simulink library "inp"  (Simulink 8.2)
settings: Solver=ode45  SolverMode=SingleTasking  StartTime=0.0  StopTime=10.0  RelTol=1e-3
undefined symbols (not defined in the file or in its folder): C_TICK_TIME_S, ENUM_FAULTSTATE_FAIL, ENUM_FAULTSTATE_INDETERMINATE, ENUM_FAULTSTATE_PASS, ENUM_KEYSWITCH_ON, FALSE, KINP_ABS1CANCommuOk_flg_ovrdflg, KINP_ABS1CANCommuOk_flg_ovrdval, KINP_ABS1CANPeriodTime_s, KINP_ABS1CANRolCntOk_flg_ovrdflg, KINP_ABS1CANRolCntOk_flg_ovrdval, KINP_ABS2CANChkSumOk_flg_ovrdflg, KINP_ABS2CANChkSumOk_flg_ovrdval, KINP_ABS2CANCommuOk_flg_ovrdflg, KINP_ABS2CANCommuOk_flg_ovrdval, KINP_ABS2CANPeriodTime_s, KINP_ABS2CANRolCntOk_flg_ovrdflg, KINP_ABS2CANRolCntOk_flg_ovrdval, KINP_ABS3CANCommuOk_flg_ovrdflg, KINP_ABS3CANCommuOk_flg_ovrdval, KINP_ABS4CANCommuOk_flg_ovrdflg, KINP_ABS4CANCommuOk_flg_ovrdval, KINP_ABSActive_flg_defval, KINP_ABSActive_flg_invalid, KINP_ABSActive_flg_ovrdflg, KINP_ABSActive_flg_ovrdval, KINP_ABSActive_flg_setvalid, KINP_ABSActive_flg_srvcflg, KINP_ABSActive_flg_srvcval, KINP_ABSCANAlive_flg_ovrdflg, KINP_ABSCANAlive_flg_ovrdval, KINP_ABSCANCommuOk_flg_ovrdflg, KINP_ABSCANCommuOk_flg_ovrdval, KINP_ABSCANValidOk_flg_ovrdflg, KINP_ABSCANValidOk_flg_ovrdval, KINP_ABSFault_flg_defval, KINP_ABSFault_flg_invalid, KINP_ABSFault_flg_ovrdflg, KINP_ABSFault_flg_ovrdval, KINP_ABSFault_flg_setvalid, KINP_ABSFault_flg_srvcflg, KINP_ABSFault_flg_srvcval, KINP_AC1CANCommuOk_flg_ovrdflg, KINP_AC1CANCommuOk_flg_ovrdval, KINP_ACCANAlive_flg_ovrdflg, KINP_ACCANAlive_flg_ovrdval, KINP_ACCANCommuOk_flg_ovrdflg, KINP_ACCANCommuOk_flg_ovrdval, KINP_ACCANValidOk_flg_ovrdflg, KINP_ACCANValidOk_flg_ovrdval, KINP_ACCCancelH_flg_defval, KINP_ACCCancelH_flg_invalid, KINP_ACCCancelH_flg_ovrdflg, KINP_ACCCancelH_flg_ovrdval, KINP_ACCCancelH_flg_setvalid, KINP_ACCCancelH_flg_srvcflg, KINP_ACCCancelH_flg_srvcval, KINP_AccelPedalPosn_pct_defval, KINP_AccelPedalPosn_pct_gain, KINP_AccelPedalPosn_pct_invalid ... (+1866)
libraries: inp x192, hcu_lib x34, simulink x33
(part 18 of 21)

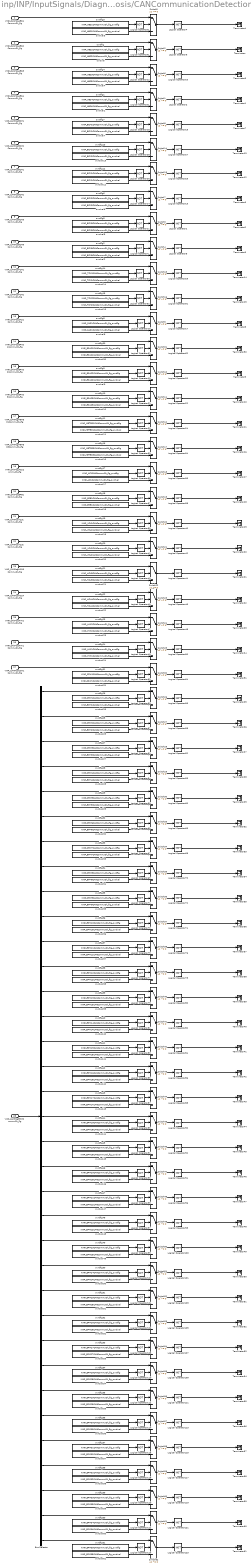
== system inp/INP/InputSignals/Diagnosis/CANCommunicationDetection_byID ==
  VINP_CANMsgABS1CommuOk_flg : Inport
  VINP_CANMsgABS2CommuOk_flg : Inport  Port=2
  VINP_CANMsgABS3CommuOk_flg : Inport  Port=3
  VINP_CANMsgABS4CommuOk_flg : Inport  Port=4
  VINP_CANMsgBCM1CommuOk_flg : Inport  Port=5
  VINP_CANMsgBCM2CommuOk_flg : Inport  Port=6
  VINP_CANMsgECM1CommuOk_flg : Inport  Port=7
  VINP_CANMsgECM2CommuOk_flg : Inport  Port=8
  VINP_CANMsgECM3CommuOk_flg : Inport  Port=9
  VINP_CANMsgECM9CommuOk_flg : Inport  Port=10
  VINP_CANMsgTCM1CommuOk_flg : Inport  Port=11
  VINP_CANMsgTCM2CommuOk_flg : Inport  Port=12
  VINP_CANMsgSAS1CommuOk_flg : Inport  Port=13
  VINP_CANMsgEPAS1CommuOk_flg : Inport  Port=14
  VINP_CANMsgEPAS2CommuOk_flg : Inport  Port=15
  VINP_CANMsgEPAS3CommuOk_flg : Inport  Port=16
  VINP_CANMsgMETER1CommuOk_flg : Inport  Port=17
  VINP_CANMsgMETER3CommuOk_flg : Inport  Port=18
  VINP_CANMsgAC1CommuOk_flg : Inport  Port=19
  VINP_CANMsgEPB1CommuOk_flg : Inport  Port=20
  VINP_CANMsgVCU1CommuOk_flg : Inport  Port=21
  VINP_CANMsgVCU2CommuOk_flg : Inport  Port=22
  VINP_CANMsgHCU3CommuOk_flg : Inport  Port=23
  VINP_CANMsgHCU4CommuOk_flg : Inport  Port=24
  VINP_CANMsgHMI2CommuOk_flg : Inport  Port=25
  VINP_CANMsgHMI1CommuOk_flg : Inport  Port=26
  VINP_CANMsgCSW1CommuOk_flg : Inport  Port=27
  VINP_CANMsgEPMCommuOk_flg : Inport  Port=28
  Bus Selector : BusSelector  OutputSignals=VINP_CANMsgEPM1CommuOk_flg,VINP_CANMsgEPM2CommuOk_flg,VINP_CANMsgEPM3CommuOk_flg,VINP_CANMsgEPM4CommuOk_flg,VINP_CANMsgEPM5CommuOk_flg,VINP_CANMsgEPM6CommuOk_flg,VINP_CANMsgEPM7CommuOk_flg,VINP_CANMsgEPM8CommuOk_flg,VINP_CANMsgEPM9CommuOk_flg,VINP_CANMsgEPM10CommuOk_flg,VINP_CANMsgEPM11CommuOk_flg,VINP_CANMsgEPM12CommuOk_flg,VINP_CANMsgEPM13CommuOk_flg,VINP_CANMsgEPM14CommuOk_flg,VINP_CANMsgEPM15CommuOk_flg,VINP_CANMsgEPM16CommuOk_flg,VINP_CANMsgEPM17CommuOk_flg,VINP_CANMsgEPM18CommuOk_flg,VINP_CANMsgEPM19CommuOk_flg,VINP_CANMsgEPM20CommuOk_flg,VINP_CANMsgEPM21CommuOk_flg,VINP_CANMsgEPM22CommuOk_flg,VINP_CANMsgEPM23CommuOk_flg,VINP_CANMsgEPM24CommuOk_flg,VINP_CANMsgEPM25CommuOk_flg,VINP_CANMsgEPM26CommuOk_flg,VINP_CANMsgEPM27CommuOk_flg,VINP_CANMsgEPM28CommuOk_flg,VINP_CANMsgEPM29CommuOk_flg,VINP_CANMsgEPM30CommuOk_flg,VINP_CANMsgEPM31CommuOk_flg,VINP_CANMsgEPM32CommuOk_flg,VINP_CANMsgEPM33CommuOk_flg,VINP_CANMsgEPM34CommuOk_flg,VINP_CANMsgEPM35CommuOk_flg
  Logical Operator{1..124} (124 alike) : Logic  Operator=NOT  AllPortsSameDT=off  OutDataTypeStr=boolean
  Switch{1..62} (62 alike) : Switch  Criteria=u2 ~= 0
  Terminator{,1..61} (62 alike) : Terminator
  ovrdflg : Constant  Value=KINP_ABS2CANCommuOk_flg_ovrdflg
  ovrdflg1 : Constant  Value=KINP_ABS4CANCommuOk_flg_ovrdflg
  ovrdflg10 : Constant  Value=KINP_EPAS3CANCommuOk_flg_ovrdflg
  ovrdflg11 : Constant  Value=KINP_METER1CANCommuOk_flg_ovrdflg
  ovrdflg12 : Constant  Value=KINP_ECM1CANCommuOk_flg_ovrdflg
  ovrdflg13 : Constant  Value=KINP_BCM2CANCommuOk_flg_ovrdflg
  ovrdflg14 : Constant  Value=KINP_TCM1CANCommuOk_flg_ovrdflg
  ovrdflg15 : Constant  Value=KINP_TCM2CANCommuOk_flg_ovrdflg
  ovrdflg16 : Constant  Value=KINP_METER3CANCommuOk_flg_ovrdflg
  ovrdflg17 : Constant  Value=KINP_AC1CANCommuOk_flg_ovrdflg
  ovrdflg18 : Constant  Value=KINP_EPB1CANCommuOk_flg_ovrdflg
  ovrdflg19 : Constant  Value=KINP_VCU1CANCommuOk_flg_ovrdflg
  ovrdflg2 : Constant  Value=KINP_ECM9CANCommuOk_flg_ovrdflg
  ovrdflg20 : Constant  Value=KINP_VCU2CANCommuOk_flg_ovrdflg
  ovrdflg21 : Constant  Value=KINP_HCU3CANCommuOk_flg_ovrdflg
  ovrdflg22 : Constant  Value=KINP_HCU4CANCommuOk_flg_ovrdflg
  ovrdflg23 : Constant  Value=KINP_HMI2CANCommuOk_flg_ovrdflg
  ovrdflg24 : Constant  Value=KINP_HMI1CANCommuOk_flg_ovrdflg
  ovrdflg25 : Constant  Value=KINP_EPM2CANCommuOk_flg_ovrdflg
  ovrdflg26 : Constant  Value=KINP_EPM1CANCommuOk_flg_ovrdflg
  ovrdflg27 : Constant  Value=KINP_EPM3CANCommuOk_flg_ovrdflg
  ovrdflg28 : Constant  Value=KINP_EPM4CANCommuOk_flg_ovrdflg
  ovrdflg29 : Constant  Value=KINP_EPM5CANCommuOk_flg_ovrdflg
  ovrdflg3 : Constant  Value=KINP_ABS1CANCommuOk_flg_ovrdflg
  ovrdflg30 : Constant  Value=KINP_EPAS1CANCommuOk_flg_ovrdflg
  ovrdflg31 : Constant  Value=KINP_CSW1CANCommuOk_flg_ovrdflg
  ovrdflg32 : Constant  Value=KINP_EPM6CANCommuOk_flg_ovrdflg
  ovrdflg33 : Constant  Value=KINP_EPM7CANCommuOk_flg_ovrdflg
  ovrdflg34 : Constant  Value=KINP_EPM8CANCommuOk_flg_ovrdflg
  ovrdflg35 : Constant  Value=KINP_EPM9CANCommuOk_flg_ovrdflg
  ovrdflg36 : Constant  Value=KINP_EPM10CANCommuOk_flg_ovrdflg
  ovrdflg37 : Constant  Value=KINP_EPM11CANCommuOk_flg_ovrdflg
  ovrdflg38 : Constant  Value=KINP_EPM12CANCommuOk_flg_ovrdflg
  ovrdflg39 : Constant  Value=KINP_EPM13CANCommuOk_flg_ovrdflg
  ovrdflg4 : Constant  Value=KINP_ABS3CANCommuOk_flg_ovrdflg
  ovrdflg40 : Constant  Value=KINP_EPM14CANCommuOk_flg_ovrdflg
  ovrdflg41 : Constant  Value=KINP_EPM15CANCommuOk_flg_ovrdflg
  ovrdflg42 : Constant  Value=KINP_EPM16CANCommuOk_flg_ovrdflg
  ovrdflg43 : Constant  Value=KINP_EPM17CANCommuOk_flg_ovrdflg
  ovrdflg44 : Constant  Value=KINP_EPM18CANCommuOk_flg_ovrdflg
  ovrdflg45 : Constant  Value=KINP_EPM19CANCommuOk_flg_ovrdflg
  ovrdflg46 : Constant  Value=KINP_EPM20CANCommuOk_flg_ovrdflg
  ovrdflg47 : Constant  Value=KINP_EPM21CANCommuOk_flg_ovrdflg
  ovrdflg48 : Constant  Value=KINP_EPM22CANCommuOk_flg_ovrdflg
  ovrdflg49 : Constant  Value=KINP_EPM23CANCommuOk_flg_ovrdflg
  ovrdflg5 : Constant  Value=KINP_ECM3CANCommuOk_flg_ovrdflg
  ovrdflg50 : Constant  Value=KINP_EPM24CANCommuOk_flg_ovrdflg
  ovrdflg51 : Constant  Value=KINP_EPM25CANCommuOk_flg_ovrdflg
  ovrdflg52 : Constant  Value=KINP_EPM26CANCommuOk_flg_ovrdflg
  ovrdflg53 : Constant  Value=KINP_EPM27CANCommuOk_flg_ovrdflg
  ovrdflg54 : Constant  Value=KINP_EPM28CANCommuOk_flg_ovrdflg
  ovrdflg55 : Constant  Value=KINP_EPM29CANCommuOk_flg_ovrdflg
  ovrdflg56 : Constant  Value=KINP_EPM30CANCommuOk_flg_ovrdflg
  ovrdflg57 : Constant  Value=KINP_EPM31CANCommuOk_flg_ovrdflg
  ovrdflg58 : Constant  Value=KINP_EPM32CANCommuOk_flg_ovrdflg
  ovrdflg59 : Constant  Value=KINP_EPM33CANCommuOk_flg_ovrdflg
  ovrdflg6 : Constant  Value=KINP_ECM2CANCommuOk_flg_ovrdflg
  ovrdflg60 : Constant  Value=KINP_EPM34CANCommuOk_flg_ovrdflg
  ovrdflg61 : Constant  Value=KINP_EPM35CANCommuOk_flg_ovrdflg
  ovrdflg7 : Constant  Value=KINP_BCM1CANCommuOk_flg_ovrdflg
  ovrdflg8 : Constant  Value=KINP_SAS1CANCommuOk_flg_ovrdflg
  ovrdflg9 : Constant  Value=KINP_EPAS2CANCommuOk_flg_ovrdflg
  ovrdval : Constant  Value=KINP_ABS2CANCommuOk_flg_ovrdval
  ovrdval1 : Constant  Value=KINP_ABS4CANCommuOk_flg_ovrdval
  ovrdval10 : Constant  Value=KINP_EPAS3CANCommuOk_flg_ovrdval
  ovrdval11 : Constant  Value=KINP_METER1CANCommuOk_flg_ovrdval
  ovrdval12 : Constant  Value=KINP_ECM1CANCommuOk_flg_ovrdval
  ovrdval13 : Constant  Value=KINP_BCM2CANCommuOk_flg_ovrdval
  ovrdval14 : Constant  Value=KINP_TCM1CANCommuOk_flg_ovrdval
  ovrdval15 : Constant  Value=KINP_TCM2CANCommuOk_flg_ovrdval
  ovrdval16 : Constant  Value=KINP_METER3CANCommuOk_flg_ovrdval
  ovrdval17 : Constant  Value=KINP_AC1CANCommuOk_flg_ovrdval
  ovrdval18 : Constant  Value=KINP_EPB1CANCommuOk_flg_ovrdval
  ovrdval19 : Constant  Value=KINP_VCU1CANCommuOk_flg_ovrdval
  ovrdval2 : Constant  Value=KINP_ECM9CANCommuOk_flg_ovrdval
  ovrdval20 : Constant  Value=KINP_VCU2CANCommuOk_flg_ovrdval
  ovrdval21 : Constant  Value=KINP_HCU3CANCommuOk_flg_ovrdval
  ovrdval22 : Constant  Value=KINP_HCU4CANCommuOk_flg_ovrdval
  ovrdval23 : Constant  Value=KINP_HMI2CANCommuOk_flg_ovrdval
  ovrdval24 : Constant  Value=KINP_HMI1CANCommuOk_flg_ovrdval
  ovrdval25 : Constant  Value=KINP_EPM2CANCommuOk_flg_ovrdval
  ovrdval26 : Constant  Value=KINP_EPM1CANCommuOk_flg_ovrdval
  ovrdval27 : Constant  Value=KINP_EPM3CANCommuOk_flg_ovrdval
  ovrdval28 : Constant  Value=KINP_EPM4CANCommuOk_flg_ovrdval
  ovrdval29 : Constant  Value=KINP_EPM5CANCommuOk_flg_ovrdval
  ovrdval3 : Constant  Value=KINP_ABS1CANCommuOk_flg_ovrdval
  ovrdval30 : Constant  Value=KINP_EPAS1CANCommuOk_flg_ovrdval
  ovrdval31 : Constant  Value=KINP_CSW1CANCommuOk_flg_ovrdval
  ovrdval32 : Constant  Value=KINP_EPM6CANCommuOk_flg_ovrdval
  ovrdval33 : Constant  Value=KINP_EPM7CANCommuOk_flg_ovrdval
  ovrdval34 : Constant  Value=KINP_EPM8CANCommuOk_flg_ovrdval
  ovrdval35 : Constant  Value=KINP_EPM9CANCommuOk_flg_ovrdval
  ovrdval36 : Constant  Value=KINP_EPM10CANCommuOk_flg_ovrdval
  ovrdval37 : Constant  Value=KINP_EPM11CANCommuOk_flg_ovrdval
  ovrdval38 : Constant  Value=KINP_EPM12CANCommuOk_flg_ovrdval
  ovrdval39 : Constant  Value=KINP_EPM13CANCommuOk_flg_ovrdval
  ovrdval4 : Constant  Value=KINP_ABS3CANCommuOk_flg_ovrdval
  ovrdval40 : Constant  Value=KINP_EPM14CANCommuOk_flg_ovrdval
  ovrdval41 : Constant  Value=KINP_EPM15CANCommuOk_flg_ovrdval
  ovrdval42 : Constant  Value=KINP_EPM16CANCommuOk_flg_ovrdval
  ovrdval43 : Constant  Value=KINP_EPM17CANCommuOk_flg_ovrdval
  ovrdval44 : Constant  Value=KINP_EPM18CANCommuOk_flg_ovrdval
  ovrdval45 : Constant  Value=KINP_EPM19CANCommuOk_flg_ovrdval
  ovrdval46 : Constant  Value=KINP_EPM20CANCommuOk_flg_ovrdval
  ovrdval47 : Constant  Value=KINP_EPM21CANCommuOk_flg_ovrdval
  ovrdval48 : Constant  Value=KINP_EPM22CANCommuOk_flg_ovrdval
  ovrdval49 : Constant  Value=KINP_EPM23CANCommuOk_flg_ovrdval
  ovrdval5 : Constant  Value=KINP_ECM3CANCommuOk_flg_ovrdval
  ovrdval50 : Constant  Value=KINP_EPM24CANCommuOk_flg_ovrdval
  ovrdval51 : Constant  Value=KINP_EPM25CANCommuOk_flg_ovrdval
  ovrdval52 : Constant  Value=KINP_EPM26CANCommuOk_flg_ovrdval
  ovrdval53 : Constant  Value=KINP_EPM27CANCommuOk_flg_ovrdval
  ovrdval54 : Constant  Value=KINP_EPM28CANCommuOk_flg_ovrdval
  ovrdval55 : Constant  Value=KINP_EPM29CANCommuOk_flg_ovrdval
  ovrdval56 : Constant  Value=KINP_EPM30CANCommuOk_flg_ovrdval
  ovrdval57 : Constant  Value=KINP_EPM31CANCommuOk_flg_ovrdval
  ovrdval58 : Constant  Value=KINP_EPM32CANCommuOk_flg_ovrdval
  ovrdval59 : Constant  Value=KINP_EPM33CANCommuOk_flg_ovrdval
  ovrdval6 : Constant  Value=KINP_ECM2CANCommuOk_flg_ovrdval
  ovrdval60 : Constant  Value=KINP_EPM34CANCommuOk_flg_ovrdval
  ovrdval61 : Constant  Value=KINP_EPM35CANCommuOk_flg_ovrdval
  ovrdval7 : Constant  Value=KINP_BCM1CANCommuOk_flg_ovrdval
  ovrdval8 : Constant  Value=KINP_SAS1CANCommuOk_flg_ovrdval
  ovrdval9 : Constant  Value=KINP_EPAS2CANCommuOk_flg_ovrdval
  wires:
  Logical Operator1/1 -> Terminator2/1  "VINP_ECM3CANCommuFault_flg"
  Logical Operator6/1 -> Terminator/1  "VINP_ABS2CANCommuFault_flg"
  Logical Operator11/1 -> Switch3/2
  Logical Operator8/1 -> Switch2/2
  ovrdval5/1 -> Switch3/3
  ovrdflg5/1 -> Logical Operator11/1
  ovrdval/1 -> Switch2/3
  ovrdflg/1 -> Logical Operator8/1
  Switch3/1 -> Logical Operator1/1
  Switch2/1 -> Logical Operator6/1
  VINP_CANMsgABS2CommuOk_flg/1 -> Switch2/1
  VINP_CANMsgECM3CommuOk_flg/1 -> Switch3/1
  Logical Operator2/1 -> Terminator1/1  "VINP_ABS4CANCommuFault_flg"
  Logical Operator3/1 -> Switch1/2
  ovrdval1/1 -> Switch1/3
  ovrdflg1/1 -> Logical Operator3/1
  Switch1/1 -> Logical Operator2/1
  VINP_CANMsgABS4CommuOk_flg/1 -> Switch1/1
  Logical Operator5/1 -> Terminator3/1  "VINP_ECM9CANCommuFault_flg"
  Logical Operator7/1 -> Switch4/2
  ovrdval2/1 -> Switch4/3
  ovrdflg2/1 -> Logical Operator7/1
  Switch4/1 -> Logical Operator5/1
  VINP_CANMsgECM9CommuOk_flg/1 -> Switch4/1
  Logical Operator17/1 -> Terminator8/1  "VINP_SAS1CANCommuFault_flg"
  Logical Operator16/1 -> Switch9/2
  ovrdval8/1 -> Switch9/3
  ovrdflg8/1 -> Logical Operator16/1
  Switch9/1 -> Logical Operator17/1
  VINP_CANMsgSAS1CommuOk_flg/1 -> Switch9/1
  Logical Operator20/1 -> Terminator9/1  "VINP_EPAS2CANCommuFault_flg"
  Logical Operator19/1 -> Switch10/2
  ovrdval9/1 -> Switch10/3
  ovrdflg9/1 -> Logical Operator19/1
  Switch10/1 -> Logical Operator20/1
  VINP_CANMsgEPAS2CommuOk_flg/1 -> Switch10/1
  Logical Operator22/1 -> Terminator10/1  "VINP_EPAS3CANCommuFault_flg"
  Logical Operator21/1 -> Switch11/2
  ovrdval10/1 -> Switch11/3
  ovrdflg10/1 -> Logical Operator21/1
  Switch11/1 -> Logical Operator22/1
  VINP_CANMsgEPAS3CommuOk_flg/1 -> Switch11/1
  Logical Operator24/1 -> Terminator11/1  "VINP_METER1CANCommuFault_flg"
  Logical Operator23/1 -> Switch12/2
  ovrdval11/1 -> Switch12/3
  ovrdflg11/1 -> Logical Operator23/1
  Switch12/1 -> Logical Operator24/1
  VINP_CANMsgMETER1CommuOk_flg/1 -> Switch12/1
  Logical Operator25/1 -> Terminator12/1  "VINP_ECM1CANCommuFault_flg"
  Logical Operator26/1 -> Switch13/2
  ovrdval12/1 -> Switch13/3
  ovrdflg12/1 -> Logical Operator26/1
  Switch13/1 -> Logical Operator25/1
  VINP_CANMsgECM1CommuOk_flg/1 -> Switch13/1
  Logical Operator4/1 -> Terminator4/1  "VINP_ABS1CANCommuFault_flg"
  Logical Operator9/1 -> Switch5/2
  ovrdval3/1 -> Switch5/3
  ovrdflg3/1 -> Logical Operator9/1
  Switch5/1 -> Logical Operator4/1
  VINP_CANMsgABS1CommuOk_flg/1 -> Switch5/1
  Logical Operator10/1 -> Terminator5/1  "VINP_ABS3CANCommuFault_flg"
  Logical Operator12/1 -> Switch6/2
  ovrdval4/1 -> Switch6/3
  ovrdflg4/1 -> Logical Operator12/1
  Switch6/1 -> Logical Operator10/1
  VINP_CANMsgABS3CommuOk_flg/1 -> Switch6/1
  Logical Operator13/1 -> Terminator6/1  "VINP_ECM2CANCommuFault_flg"
  Logical Operator14/1 -> Switch7/2
  ovrdval6/1 -> Switch7/3
  ovrdflg6/1 -> Logical Operator14/1
  Switch7/1 -> Logical Operator13/1
  VINP_CANMsgECM2CommuOk_flg/1 -> Switch7/1
  Logical Operator15/1 -> Terminator7/1  "VINP_BCM1CANCommuFault_flg"
  Logical Operator18/1 -> Switch8/2
  ovrdval7/1 -> Switch8/3
  ovrdflg7/1 -> Logical Operator18/1
  Switch8/1 -> Logical Operator15/1
  VINP_CANMsgBCM1CommuOk_flg/1 -> Switch8/1
  Logical Operator27/1 -> Terminator13/1  "VINP_BCM2CANCommuFault_flg"
  Logical Operator28/1 -> Switch14/2
  ovrdval13/1 -> Switch14/3
  ovrdflg13/1 -> Logical Operator28/1
  Switch14/1 -> Logical Operator27/1
  VINP_CANMsgBCM2CommuOk_flg/1 -> Switch14/1
  Logical Operator29/1 -> Terminator14/1  "VINP_TCM1CANCommuFault_flg"
  Logical Operator30/1 -> Switch15/2
  ovrdval14/1 -> Switch15/3
  ovrdflg14/1 -> Logical Operator30/1
  Switch15/1 -> Logical Operator29/1
  VINP_CANMsgTCM1CommuOk_flg/1 -> Switch15/1
  Logical Operator31/1 -> Terminator15/1  "VINP_TCM2CANCommuFault_flg"
  Logical Operator32/1 -> Switch16/2
  ovrdval15/1 -> Switch16/3
  ovrdflg15/1 -> Logical Operator32/1
  Switch16/1 -> Logical Operator31/1
  VINP_CANMsgTCM2CommuOk_flg/1 -> Switch16/1
  Logical Operator34/1 -> Terminator16/1  "VINP_METER3CANCommuFault_flg"
  Logical Operator33/1 -> Switch17/2
  ovrdval16/1 -> Switch17/3
  ovrdflg16/1 -> Logical Operator33/1
  Switch17/1 -> Logical Operator34/1
  VINP_CANMsgMETER3CommuOk_flg/1 -> Switch17/1
  Logical Operator36/1 -> Terminator17/1  "VINP_AC1CANCommuFault_flg"
  Logical Operator35/1 -> Switch18/2
  ovrdval17/1 -> Switch18/3
  ovrdflg17/1 -> Logical Operator35/1
  Switch18/1 -> Logical Operator36/1
  VINP_CANMsgAC1CommuOk_flg/1 -> Switch18/1
  Logical Operator38/1 -> Terminator18/1  "VINP_EPB1CANCommuFault_flg"
  Logical Operator37/1 -> Switch19/2
  ovrdval18/1 -> Switch19/3
  ovrdflg18/1 -> Logical Operator37/1
  Switch19/1 -> Logical Operator38/1
  VINP_CANMsgEPB1CommuOk_flg/1 -> Switch19/1
  Logical Operator40/1 -> Terminator19/1  "VINP_VCU1CANCommuFault_flg"
  Logical Operator39/1 -> Switch20/2
  ovrdval19/1 -> Switch20/3
  ovrdflg19/1 -> Logical Operator39/1
  Switch20/1 -> Logical Operator40/1
  VINP_CANMsgVCU1CommuOk_flg/1 -> Switch20/1
  Logical Operator42/1 -> Terminator20/1  "VINP_VCU2CANCommuFault_flg"
  Logical Operator41/1 -> Switch21/2
  ovrdval20/1 -> Switch21/3
  ovrdflg20/1 -> Logical Operator41/1
  Switch21/1 -> Logical Operator42/1
  VINP_CANMsgVCU2CommuOk_flg/1 -> Switch21/1
  Logical Operator44/1 -> Terminator21/1  "VINP_HCU3CANCommuFault_flg"
  Logical Operator43/1 -> Switch22/2
  ovrdval21/1 -> Switch22/3
  ovrdflg21/1 -> Logical Operator43/1
  Switch22/1 -> Logical Operator44/1
  VINP_CANMsgHCU3CommuOk_flg/1 -> Switch22/1
  Logical Operator46/1 -> Terminator22/1  "VINP_HCU4CANCommuFault_flg"
  Logical Operator45/1 -> Switch23/2
  ovrdval22/1 -> Switch23/3
  ovrdflg22/1 -> Logical Operator45/1
  Switch23/1 -> Logical Operator46/1
  VINP_CANMsgHCU4CommuOk_flg/1 -> Switch23/1
  Logical Operator48/1 -> Terminator23/1  "VINP_HMI2CANCommuFault_flg"
  Logical Operator47/1 -> Switch24/2
  ovrdval23/1 -> Switch24/3
  ovrdflg23/1 -> Logical Operator47/1
  Switch24/1 -> Logical Operator48/1
  VINP_CANMsgHMI2CommuOk_flg/1 -> Switch24/1
  Logical Operator50/1 -> Terminator24/1  "VINP_HMI1CANCommuFault_flg"
  Logical Operator49/1 -> Switch25/2
  ovrdval24/1 -> Switch25/3
  ovrdflg24/1 -> Logical Operator49/1
  Switch25/1 -> Logical Operator50/1
  VINP_CANMsgHMI1CommuOk_flg/1 -> Switch25/1
  Logical Operator56/1 -> Terminator25/1  "VINP_EPM1CANCommuFault_flg"
  Logical Operator53/1 -> Switch26/2
  ovrdval26/1 -> Switch26/3
  ovrdflg26/1 -> Logical Operator53/1
  Switch26/1 -> Logical Operator56/1
  VINP_CANMsgEPMCommuOk_flg/1 -> Bus Selector/1
  Logical Operator58/1 -> Terminator26/1  "VINP_EPM2CANCommuFault_flg"
  Logical Operator57/1 -> Switch27/2
  ovrdval25/1 -> Switch27/3
  ovrdflg25/1 -> Logical Operator57/1
  Switch27/1 -> Logical Operator58/1
  Logical Operator52/1 -> Terminator27/1  "VINP_EPM3CANCommuFault_flg"
  Logical Operator51/1 -> Switch28/2
  ovrdval27/1 -> Switch28/3
  ovrdflg27/1 -> Logical Operator51/1
  Switch28/1 -> Logical Operator52/1
  Logical Operator55/1 -> Terminator28/1  "VINP_EPM4CANCommuFault_flg"
  Logical Operator54/1 -> Switch29/2
  ovrdval28/1 -> Switch29/3
  ovrdflg28/1 -> Logical Operator54/1
  Switch29/1 -> Logical Operator55/1
  Logical Operator60/1 -> Terminator29/1  "VINP_EPM5CANCommuFault_flg"
  Logical Operator59/1 -> Switch30/2
  ovrdval29/1 -> Switch30/3
  ovrdflg29/1 -> Logical Operator59/1
  Switch30/1 -> Logical Operator60/1
  Bus Selector/5 -> Switch30/1  "<VINP_CANMsgEPM5CommuOk_flg>"
  Logical Operator62/1 -> Terminator30/1  "VINP_EPAS1CANCommuFault_flg"
  Logical Operator61/1 -> Switch31/2
  ovrdval30/1 -> Switch31/3
  ovrdflg30/1 -> Logical Operator61/1
  Switch31/1 -> Logical Operator62/1
  VINP_CANMsgEPAS1CommuOk_flg/1 -> Switch31/1
  Logical Operator64/1 -> Terminator31/1  "VINP_CSW1CANCommuFault_flg"
  Logical Operator63/1 -> Switch32/2
  ovrdval31/1 -> Switch32/3
  ovrdflg31/1 -> Logical Operator63/1
  Switch32/1 -> Logical Operator64/1
  VINP_CANMsgCSW1CommuOk_flg/1 -> Switch32/1
  Bus Selector/1 -> Switch26/1  "<VINP_CANMsgEPM1CommuOk_flg>"
  Bus Selector/2 -> Switch27/1  "<VINP_CANMsgEPM2CommuOk_flg>"
  Bus Selector/3 -> Switch28/1  "<VINP_CANMsgEPM3CommuOk_flg>"
  Bus Selector/4 -> Switch29/1  "<VINP_CANMsgEPM4CommuOk_flg>"
  Logical Operator66/1 -> Terminator32/1  "VINP_EPM6CANCommuFault_flg"
  Logical Operator65/1 -> Switch33/2
  ovrdval32/1 -> Switch33/3
  ovrdflg32/1 -> Logical Operator65/1
  Switch33/1 -> Logical Operator66/1
  Bus Selector/6 -> Switch33/1  "<VINP_CANMsgEPM6CommuOk_flg>"
  Logical Operator68/1 -> Terminator33/1  "VINP_EPM7CANCommuFault_flg"
  Logical Operator67/1 -> Switch34/2
  ovrdval33/1 -> Switch34/3
  ovrdflg33/1 -> Logical Operator67/1
  Switch34/1 -> Logical Operator68/1
  Bus Selector/7 -> Switch34/1  "<VINP_CANMsgEPM7CommuOk_flg>"
  Logical Operator70/1 -> Terminator34/1  "VINP_EPM8CANCommuFault_flg"
  Logical Operator69/1 -> Switch35/2
  ovrdval34/1 -> Switch35/3
  ovrdflg34/1 -> Logical Operator69/1
  Switch35/1 -> Logical Operator70/1
  Bus Selector/8 -> Switch35/1  "<VINP_CANMsgEPM8CommuOk_flg>"
  Logical Operator72/1 -> Terminator35/1  "VINP_EPM9CANCommuFault_flg"
  Logical Operator71/1 -> Switch36/2
  ovrdval35/1 -> Switch36/3
  ovrdflg35/1 -> Logical Operator71/1
  Switch36/1 -> Logical Operator72/1
  Bus Selector/9 -> Switch36/1  "<VINP_CANMsgEPM9CommuOk_flg>"
  Logical Operator74/1 -> Terminator36/1  "VINP_EPM10CANCommuFault_flg"
  Logical Operator73/1 -> Switch37/2
  ovrdval36/1 -> Switch37/3
  ovrdflg36/1 -> Logical Operator73/1
  Switch37/1 -> Logical Operator74/1
  Bus Selector/10 -> Switch37/1  "<VINP_CANMsgEPM10CommuOk_flg>"
  Logical Operator76/1 -> Terminator37/1  "VINP_EPM11CANCommuFault_flg"
  Logical Operator75/1 -> Switch38/2
  ovrdval37/1 -> Switch38/3
  ovrdflg37/1 -> Logical Operator75/1
  Switch38/1 -> Logical Operator76/1
  Bus Selector/11 -> Switch38/1  "<VINP_CANMsgEPM11CommuOk_flg>"
  Logical Operator78/1 -> Terminator38/1  "VINP_EPM12CANCommuFault_flg"
  Logical Operator77/1 -> Switch39/2
  ovrdval38/1 -> Switch39/3
  ovrdflg38/1 -> Logical Operator77/1
  Switch39/1 -> Logical Operator78/1
  Bus Selector/12 -> Switch39/1  "<VINP_CANMsgEPM12CommuOk_flg>"
  Logical Operator80/1 -> Terminator39/1  "VINP_EPM13CANCommuFault_flg"
  Logical Operator79/1 -> Switch40/2
  ovrdval39/1 -> Switch40/3
  ovrdflg39/1 -> Logical Operator79/1
  Switch40/1 -> Logical Operator80/1
  Bus Selector/13 -> Switch40/1  "<VINP_CANMsgEPM13CommuOk_flg>"
  Logical Operator82/1 -> Terminator40/1  "VINP_EPM14CANCommuFault_flg"
  Logical Operator81/1 -> Switch41/2
  ovrdval40/1 -> Switch41/3
  ovrdflg40/1 -> Logical Operator81/1
  Switch41/1 -> Logical Operator82/1
  Bus Selector/14 -> Switch41/1  "<VINP_CANMsgEPM14CommuOk_flg>"
  Logical Operator84/1 -> Terminator41/1  "VINP_EPM15CANCommuFault_flg"
  Logical Operator83/1 -> Switch42/2
  ovrdval41/1 -> Switch42/3
  ovrdflg41/1 -> Logical Operator83/1
  Switch42/1 -> Logical Operator84/1
  Bus Selector/15 -> Switch42/1  "<VINP_CANMsgEPM15CommuOk_flg>"
  Logical Operator86/1 -> Terminator42/1  "VINP_EPM16CANCommuFault_flg"
  Logical Operator85/1 -> Switch43/2
  ovrdval42/1 -> Switch43/3
  ovrdflg42/1 -> Logical Operator85/1
  Switch43/1 -> Logical Operator86/1
  Bus Selector/16 -> Switch43/1  "<VINP_CANMsgEPM16CommuOk_flg>"
  Logical Operator88/1 -> Terminator43/1  "VINP_EPM17CANCommuFault_flg"
  Logical Operator87/1 -> Switch44/2
  ovrdval43/1 -> Switch44/3
  ovrdflg43/1 -> Logical Operator87/1
  Switch44/1 -> Logical Operator88/1
  Bus Selector/17 -> Switch44/1  "<VINP_CANMsgEPM17CommuOk_flg>"
  Logical Operator90/1 -> Terminator44/1  "VINP_EPM18CANCommuFault_flg"
  Logical Operator89/1 -> Switch45/2
  ovrdval44/1 -> Switch45/3
  ovrdflg44/1 -> Logical Operator89/1
  Switch45/1 -> Logical Operator90/1
  Bus Selector/18 -> Switch45/1  "<VINP_CANMsgEPM18CommuOk_flg>"
  Logical Operator92/1 -> Terminator45/1  "VINP_EPM19CANCommuFault_flg"
  Logical Operator91/1 -> Switch46/2
  ovrdval45/1 -> Switch46/3
  ovrdflg45/1 -> Logical Operator91/1
  Switch46/1 -> Logical Operator92/1
  Bus Selector/19 -> Switch46/1  "<VINP_CANMsgEPM19CommuOk_flg>"
  Logical Operator94/1 -> Terminator46/1  "VINP_EPM20CANCommuFault_flg"
  Logical Operator93/1 -> Switch47/2
  ovrdval46/1 -> Switch47/3
  ovrdflg46/1 -> Logical Operator93/1
  Switch47/1 -> Logical Operator94/1
  Bus Selector/20 -> Switch47/1  "<VINP_CANMsgEPM20CommuOk_flg>"
  Logical Operator96/1 -> Terminator47/1  "VINP_EPM21CANCommuFault_flg"
  Logical Operator95/1 -> Switch48/2
  ovrdval47/1 -> Switch48/3
  ovrdflg47/1 -> Logical Operator95/1
  Switch48/1 -> Logical Operator96/1
  Bus Selector/21 -> Switch48/1  "<VINP_CANMsgEPM21CommuOk_flg>"
  Logical Operator98/1 -> Terminator48/1  "VINP_EPM22CANCommuFault_flg"
  Logical Operator97/1 -> Switch49/2
  ovrdval48/1 -> Switch49/3
  ovrdflg48/1 -> Logical Operator97/1
  Switch49/1 -> Logical Operator98/1
  Bus Selector/22 -> Switch49/1  "<VINP_CANMsgEPM22CommuOk_flg>"
  Logical Operator100/1 -> Terminator49/1  "VINP_EPM23CANCommuFault_flg"
  Logical Operator99/1 -> Switch50/2
  ovrdval49/1 -> Switch50/3
  ovrdflg49/1 -> Logical Operator99/1
  Switch50/1 -> Logical Operator100/1
  Bus Selector/23 -> Switch50/1  "<VINP_CANMsgEPM23CommuOk_flg>"
  Logical Operator101/1 -> Terminator50/1  "VINP_EPM24CANCommuFault_flg"
  Logical Operator102/1 -> Switch51/2
  ovrdval50/1 -> Switch51/3
  ovrdflg50/1 -> Logical Operator102/1
  Switch51/1 -> Logical Operator101/1
  Bus Selector/24 -> Switch51/1  "<VINP_CANMsgEPM24CommuOk_flg>"
  Logical Operator103/1 -> Terminator51/1  "VINP_EPM25CANCommuFault_flg"
  Logical Operator104/1 -> Switch52/2
  ovrdval51/1 -> Switch52/3
  ovrdflg51/1 -> Logical Operator104/1
  Switch52/1 -> Logical Operator103/1
  Bus Selector/25 -> Switch52/1  "<VINP_CANMsgEPM25CommuOk_flg>"
  Logical Operator105/1 -> Terminator52/1  "VINP_EPM26CANCommuFault_flg"
  Logical Operator106/1 -> Switch53/2
  ovrdval52/1 -> Switch53/3
  ovrdflg52/1 -> Logical Operator106/1
  Switch53/1 -> Logical Operator105/1
  Bus Selector/26 -> Switch53/1  "<VINP_CANMsgEPM26CommuOk_flg>"
  Logical Operator107/1 -> Terminator53/1  "VINP_EPM27CANCommuFault_flg"
  Logical Operator108/1 -> Switch54/2
  ovrdval53/1 -> Switch54/3
  ovrdflg53/1 -> Logical Operator108/1
  Switch54/1 -> Logical Operator107/1
  Bus Selector/27 -> Switch54/1  "<VINP_CANMsgEPM27CommuOk_flg>"
  Logical Operator109/1 -> Terminator54/1  "VINP_EPM28CANCommuFault_flg"
  Logical Operator110/1 -> Switch55/2
  ovrdval54/1 -> Switch55/3
  ovrdflg54/1 -> Logical Operator110/1
  Switch55/1 -> Logical Operator109/1
  Bus Selector/28 -> Switch55/1  "<VINP_CANMsgEPM28CommuOk_flg>"
  Logical Operator111/1 -> Terminator55/1  "VINP_EPM29CANCommuFault_flg"
  Logical Operator112/1 -> Switch56/2
  ovrdval55/1 -> Switch56/3
  ovrdflg55/1 -> Logical Operator112/1
  Switch56/1 -> Logical Operator111/1
  Bus Selector/29 -> Switch56/1  "<VINP_CANMsgEPM29CommuOk_flg>"
  Logical Operator113/1 -> Terminator56/1  "VINP_EPM30CANCommuFault_flg"
  Logical Operator114/1 -> Switch57/2
  ovrdval56/1 -> Switch57/3
  ovrdflg56/1 -> Logical Operator114/1
  Switch57/1 -> Logical Operator113/1
  Bus Selector/30 -> Switch57/1  "<VINP_CANMsgEPM30CommuOk_flg>"
  Logical Operator115/1 -> Terminator57/1  "VINP_EPM31CANCommuFault_flg"
  Logical Operator116/1 -> Switch58/2
  ovrdval57/1 -> Switch58/3
  ovrdflg57/1 -> Logical Operator116/1
  Switch58/1 -> Logical Operator115/1
  Bus Selector/31 -> Switch58/1  "<VINP_CANMsgEPM31CommuOk_flg>"
  Logical Operator117/1 -> Terminator58/1  "VINP_EPM32CANCommuFault_flg"
  Logical Operator118/1 -> Switch59/2
  ovrdval58/1 -> Switch59/3
  ovrdflg58/1 -> Logical Operator118/1
  Switch59/1 -> Logical Operator117/1
  Bus Selector/32 -> Switch59/1  "<VINP_CANMsgEPM32CommuOk_flg>"
  Logical Operator119/1 -> Terminator59/1  "VINP_EPM33CANCommuFault_flg"
  Logical Operator120/1 -> Switch60/2
  ovrdval59/1 -> Switch60/3
  ovrdflg59/1 -> Logical Operator120/1
  Switch60/1 -> Logical Operator119/1
  Bus Selector/33 -> Switch60/1  "<VINP_CANMsgEPM33CommuOk_flg>"
  Logical Operator121/1 -> Terminator60/1  "VINP_EPM34CANCommuFault_flg"
  Logical Operator122/1 -> Switch61/2
  ovrdval60/1 -> Switch61/3
  ovrdflg60/1 -> Logical Operator122/1
  Switch61/1 -> Logical Operator121/1
  Bus Selector/34 -> Switch61/1  "<VINP_CANMsgEPM34CommuOk_flg>"
  Logical Operator123/1 -> Terminator61/1  "VINP_EPM35CANCommuFault_flg"
  Logical Operator124/1 -> Switch62/2
  ovrdval61/1 -> Switch62/3
  ovrdflg61/1 -> Logical Operator124/1
  Switch62/1 -> Logical Operator123/1
  Bus Selector/35 -> Switch62/1  "<VINP_CANMsgEPM35CommuOk_flg>"

== system inp/INP/InputSignals/Diagnosis/CANInvalidDetection ==
  VINP_CANMsgABS1CommuOk_flg : Inport
  VINP_CANMsgABS2CommuOk_flg : Inport  Port=2
  VINP_CANMsgABS3CommuOk_flg : Inport  Port=3
  VINP_CANMsgABS4CommuOk_flg : Inport  Port=4
  VINP_CANMsgBCM1CommuOk_flg : Inport  Port=5
  VINP_CANMsgBCM2CommuOk_flg : Inport  Port=6
  VINP_CANMsgECM1CommuOk_flg : Inport  Port=7
  VINP_CANMsgECM2CommuOk_flg : Inport  Port=8
  VINP_CANMsgECM3CommuOk_flg : Inport  Port=9
  VINP_CANMsgECM9CommuOk_flg : Inport  Port=10
  VINP_CANMsgTCM1CommuOk_flg : Inport  Port=11
  VINP_CANMsgTCM2CommuOk_flg : Inport  Port=12
  VINP_CANMsgSAS1CommuOk_flg : Inport  Port=13
  VINP_CANMsgEPAS1CommuOk_flg : Inport  Port=14
  VINP_CANMsgEPAS2CommuOk_flg : Inport  Port=15
  VINP_CANMsgEPAS3CommuOk_flg : Inport  Port=16
  VINP_CANMsgMETER1CommuOk_flg : Inport  Port=17
  VINP_CANMsgMETER3CommuOk_flg : Inport  Port=18
  VINP_CANMsgAC1CommuOk_flg : Inport  Port=19
  VINP_CANMsgEPB1CommuOk_flg : Inport  Port=20
  VINP_CANMsgVCU1CommuOk_flg : Inport  Port=21
  VINP_CANMsgVCU2CommuOk_flg : Inport  Port=22
  VINP_CANMsgHCU3CommuOk_flg : Inport  Port=23
  VINP_CANMsgHCU4CommuOk_flg : Inport  Port=24
  VINP_CANMsgHMI2CommuOk_flg : Inport  Port=25
  VINP_CANMsgHMI1CommuOk_flg : Inport  Port=26
  VINP_CANMsgCSW1CommuOk_flg : Inport  Port=27
  VINP_CANMsgEPMCommuOk_flg : Inport  Port=28
  VINP_CamCANReceiveOk_flg : Inport  Port=29
  VINP_CANMsgECM9RolCntOk_flg : Inport  Port=30
  VINP_CANMsgABS1RolCntOk_flg : Inport  Port=31
  VINP_CANMsgABS2RolCntOk_flg : Inport  Port=32
  VINP_CANMsgSAS1RolCntOk_flg : Inport  Port=33
  VINP_CANMsgEPAS2RolCntOk_flg : Inport  Port=34
  VINP_CANMsgEPAS3RolCntOk_flg : Inport  Port=35
  VINP_CANMsgVCU1RolCntOk_flg : Inport  Port=36
  VINP_CANMsgVCU2RolCntOk_flg : Inport  Port=37
  VINP_CANMsgHCU3RolCntOk_flg : Inport  Port=38
  VINP_CANMsgABS2ChkSumOk_flg : Inport  Port=39
  VINP_CANMsgSAS1ChkSumOk_flg : Inport  Port=40
  VINP_CANMsgEPAS2ChkSumOk_flg : Inport  Port=41
  VINP_CANMsgEPAS3ChkSumOk_flg : Inport  Port=42
  VINP_CANMsgVCU1ChkSumOk_flg : Inport  Port=43
  VINP_CANMsgVCU2ChkSumOk_flg : Inport  Port=44
  Bus Selector : BusSelector  OutputSignals=VINP_CANMsgEPM1CommuOk_flg,VINP_CANMsgEPM2CommuOk_flg,VINP_CANMsgEPM3CommuOk_flg,VINP_CANMsgEPM4CommuOk_flg,VINP_CANMsgEPM5CommuOk_flg,VINP_CANMsgEPM6CommuOk_flg,VINP_CANMsgEPM7CommuOk_flg,VINP_CANMsgEPM8CommuOk_flg,VINP_CANMsgEPM9CommuOk_flg,VINP_CANMsgEPM10CommuOk_flg,VINP_CANMsgEPM11CommuOk_flg,VINP_CANMsgEPM12CommuOk_flg,VINP_CANMsgEPM13CommuOk_flg,VINP_CANMsgEPM14CommuOk_flg,VINP_CANMsgEPM15CommuOk_flg,VINP_CANMsgEPM16CommuOk_flg,VINP_CANMsgEPM17CommuOk_flg,VINP_CANMsgEPM18CommuOk_flg,VINP_CANMsgEPM19CommuOk_flg,VINP_CANMsgEPM20CommuOk_flg,VINP_CANMsgEPM21CommuOk_flg,VINP_CANMsgEPM22CommuOk_flg,VINP_CANMsgEPM23CommuOk_flg,VINP_CANMsgEPM24CommuOk_flg,VINP_CANMsgEPM25CommuOk_flg,VINP_CANMsgEPM26CommuOk_flg,VINP_CANMsgEPM27CommuOk_flg,VINP_CANMsgEPM28CommuOk_flg,VINP_CANMsgEPM29CommuOk_flg,VINP_CANMsgEPM30CommuOk_flg,VINP_CANMsgEPM31CommuOk_flg,VINP_CANMsgEPM32CommuOk_flg,VINP_CANMsgEPM33CommuOk_flg,VINP_CANMsgEPM34CommuOk_flg,VINP_CANMsgEPM35CommuOk_flg
  Bus Selector1 : BusSelector  OutputSignals=VINP_CamLeftLaneReceiveOk_flg,VINP_CamRightLaneReceiveOk_flg,VINP_CamObs1ReceiveOk_flg,VINP_CamObs2ReceiveOk_flg,VINP_CamObs3ReceiveOk_flg,VINP_CamObs4ReceiveOk_flg,VINP_CamObs5ReceiveOk_flg,VINP_CamObs6ReceiveOk_flg,VINP_CamObs7ReceiveOk_flg,VINP_CamObs8ReceiveOk_flg,VINP_CamObs9ReceiveOk_flg,VINP_CamObs10ReceiveOk_flg
  Logical Operator{1,4..6,8..12,14..15,17..19,23..24,26..27,29..32,35..36} (24 alike) : Logic  Operator=NOT  AllPortsSameDT=off  OutDataTypeStr=boolean
  Logical Operator{13,22,25,34} (4 alike) : Logic  Inputs=1  AllPortsSameDT=off  OutDataTypeStr=boolean
  Logical Operator16 : Logic  Inputs=5  AllPortsSameDT=off  OutDataTypeStr=boolean
  Logical Operator2 : Logic  AllPortsSameDT=off  OutDataTypeStr=boolean
  Logical Operator20 : Logic  Inputs=10  AllPortsSameDT=off  OutDataTypeStr=boolean
  Logical Operator21 : Logic  Inputs=7  AllPortsSameDT=off  OutDataTypeStr=boolean
  Logical Operator28 : Logic  AllPortsSameDT=off  OutDataTypeStr=boolean
  Logical Operator3 : Logic  AllPortsSameDT=off  OutDataTypeStr=boolean
  Logical Operator33 : Logic  Inputs=47  AllPortsSameDT=off  OutDataTypeStr=boolean
  Logical Operator7 : Logic  Inputs=10  AllPortsSameDT=off  OutDataTypeStr=boolean
  Switch{1..12} (12 alike) : Switch  Criteria=u2 ~= 0
  Terminator{,1..11} (12 alike) : Terminator
  ovrdflg : Constant  Value=KINP_ABSCANValidOk_flg_ovrdflg
  ovrdflg1 : Constant  Value=KINP_METERCANValidOk_flg_ovrdflg
  ovrdflg10 : Constant  Value=KINP_EPMCANValidOk_flg_ovrdflg
  ovrdflg11 : Constant  Value=KINP_CSWCANValidOk_flg_ovrdflg
  ovrdflg2 : Constant  Value=KINP_BCMCANValidOk_flg_ovrdflg
  ovrdflg3 : Constant  Value=KINP_EPSCANValidOk_flg_ovrdflg
  ovrdflg4 : Constant  Value=KINP_ACCANValidOk_flg_ovrdflg
  ovrdflg5 : Constant  Value=KINP_ECMCANValidOk_flg_ovrdflg
  ovrdflg6 : Constant  Value=KINP_EPBCANValidOk_flg_ovrdflg
  ovrdflg7 : Constant  Value=KINP_VCUCANValidOk_flg_ovrdflg
  ovrdflg8 : Constant  Value=KINP_TCMCANValidOk_flg_ovrdflg
  ovrdflg9 : Constant  Value=KINP_HMICANValidOk_flg_ovrdflg
  ovrdval : Constant  Value=KINP_ABSCANValidOk_flg_ovrdval
  ovrdval1 : Constant  Value=KINP_METERCANValidOk_flg_ovrdval
  ovrdval10 : Constant  Value=KINP_EPMCANValidOk_flg_ovrdval
  ovrdval11 : Constant  Value=KINP_CSWCANValidOk_flg_ovrdval
  ovrdval2 : Constant  Value=KINP_BCMCANValidOk_flg_ovrdval
  ovrdval3 : Constant  Value=KINP_EPSCANValidOk_flg_ovrdval
  ovrdval4 : Constant  Value=KINP_ACCANValidOk_flg_ovrdval
  ovrdval5 : Constant  Value=KINP_ECMCANValidOk_flg_ovrdval
  ovrdval6 : Constant  Value=KINP_EPBCANValidOk_flg_ovrdval
  ovrdval7 : Constant  Value=KINP_VCUCANValidOk_flg_ovrdval
  ovrdval8 : Constant  Value=KINP_TCMCANValidOk_flg_ovrdval
  ovrdval9 : Constant  Value=KINP_HMICANValidOk_flg_ovrdval
  wires:
  Logical Operator19/1 -> Terminator5/1  "VINP_EPSCANInvalidFault_flg"
  Logical Operator17/1 -> Terminator3/1  "VINP_TCMCANInvalidFault_flg"
  Logical Operator1/1 -> Terminator2/1  "VINP_ECMCANInvalidFault_flg"
  Logical Operator9/1 -> Terminator1/1  "VINP_BCMCANInvalidFault_flg"
  Logical Operator6/1 -> Terminator/1  "VINP_ABSCANInvalidFault_flg"
  Logical Operator12/1 -> Switch4/2
  Logical Operator11/1 -> Switch3/2
  Logical Operator10/1 -> Switch1/2
  Logical Operator8/1 -> Switch2/2
  Logical Operator28/1 -> Switch4/1
  Logical Operator16/1 -> Switch3/1
  Logical Operator2/1 -> Switch1/1
  Logical Operator21/1 -> Switch2/1
  ovrdval8/1 -> Switch4/3
  ovrdflg8/1 -> Logical Operator12/1
  ovrdval5/1 -> Switch3/3
  ovrdflg5/1 -> Logical Operator11/1
  ovrdval2/1 -> Switch1/3
  ovrdflg2/1 -> Logical Operator10/1
  ovrdval/1 -> Switch2/3
  ovrdflg/1 -> Logical Operator8/1
  Switch4/1 -> Logical Operator17/1
  Switch3/1 -> Logical Operator1/1
  Switch1/1 -> Logical Operator9/1
  VINP_CANMsgBCM1CommuOk_flg/1 -> Logical Operator2/1
  Switch2/1 -> Logical Operator6/1
  Logical Operator15/1 -> Switch7/2
  Logical Operator7/1 -> Switch7/1
  ovrdval3/1 -> Switch7/3
  ovrdflg3/1 -> Logical Operator15/1
  Switch7/1 -> Logical Operator19/1
  Logical Operator14/1 -> Terminator6/1  "VINP_METERCANInvalidFault_flg"
  Logical Operator5/1 -> Switch6/2
  Logical Operator3/1 -> Switch6/1
  ovrdflg1/1 -> Logical Operator5/1
  Switch6/1 -> Logical Operator14/1
  VINP_CANMsgMETER1CommuOk_flg/1 -> Logical Operator3/1
  ovrdval1/1 -> Switch6/3
  Logical Operator4/1 -> Terminator4/1  "VINP_ACCANInvalidFault_flg"
  Logical Operator18/1 -> Switch5/2
  Logical Operator13/1 -> Switch5/1
  ovrdflg4/1 -> Logical Operator18/1
  Switch5/1 -> Logical Operator4/1
  VINP_CANMsgAC1CommuOk_flg/1 -> Logical Operator13/1
  ovrdval4/1 -> Switch5/3
  Logical Operator24/1 -> Terminator7/1  "VINP_EPBCANInvalidFault_flg"
  Logical Operator23/1 -> Switch8/2
  Logical Operator22/1 -> Switch8/1
  ovrdflg6/1 -> Logical Operator23/1
  Switch8/1 -> Logical Operator24/1
  VINP_CANMsgEPB1CommuOk_flg/1 -> Logical Operator22/1
  ovrdval6/1 -> Switch8/3
  Logical Operator27/1 -> Terminator8/1  "VINP_VCUCANInvalidFault_flg"
  Logical Operator26/1 -> Switch9/2
  Logical Operator20/1 -> Switch9/1
  ovrdflg7/1 -> Logical Operator26/1
  Switch9/1 -> Logical Operator27/1
  ovrdval7/1 -> Switch9/3
  VINP_CANMsgABS1CommuOk_flg/1 -> Logical Operator21/1
  VINP_CANMsgABS2CommuOk_flg/1 -> Logical Operator21/2
  VINP_CANMsgABS3CommuOk_flg/1 -> Logical Operator21/3
  VINP_CANMsgABS4CommuOk_flg/1 -> Logical Operator21/4
  VINP_CANMsgABS1RolCntOk_flg/1 -> Logical Operator21/5
  VINP_CANMsgABS2RolCntOk_flg/1 -> Logical Operator21/6
  VINP_CANMsgABS2ChkSumOk_flg/1 -> Logical Operator21/7
  VINP_CANMsgBCM2CommuOk_flg/1 -> Logical Operator2/2
  VINP_CANMsgECM1CommuOk_flg/1 -> Logical Operator16/1
  VINP_CANMsgECM2CommuOk_flg/1 -> Logical Operator16/2
  VINP_CANMsgECM3CommuOk_flg/1 -> Logical Operator16/3
  VINP_CANMsgECM9CommuOk_flg/1 -> Logical Operator16/4
  VINP_CANMsgECM9RolCntOk_flg/1 -> Logical Operator16/5
  VINP_CANMsgTCM1CommuOk_flg/1 -> Logical Operator28/1
  VINP_CANMsgTCM2CommuOk_flg/1 -> Logical Operator28/2
  VINP_CANMsgSAS1CommuOk_flg/1 -> Logical Operator7/1
  VINP_CANMsgMETER3CommuOk_flg/1 -> Logical Operator3/2
  Logical Operator30/1 -> Terminator9/1  "VINP_HMICANInvalidFault_flg"
  Logical Operator29/1 -> Switch10/2
  Logical Operator25/1 -> Switch10/1
  ovrdflg9/1 -> Logical Operator29/1
  Switch10/1 -> Logical Operator30/1
  VINP_CANMsgHMI1CommuOk_flg/1 -> Logical Operator25/1
  ovrdval9/1 -> Switch10/3
  Logical Operator32/1 -> Terminator10/1  "VINP_EPMCANInvalidFault_flg"
  Logical Operator31/1 -> Switch11/2
  Logical Operator33/1 -> Switch11/1
  ovrdval10/1 -> Switch11/3
  ovrdflg10/1 -> Logical Operator31/1
  Switch11/1 -> Logical Operator32/1
  VINP_CANMsgVCU1CommuOk_flg/1 -> Logical Operator20/1
  VINP_CANMsgVCU2CommuOk_flg/1 -> Logical Operator20/2
  VINP_CANMsgHCU3CommuOk_flg/1 -> Logical Operator20/3
  VINP_CANMsgHCU4CommuOk_flg/1 -> Logical Operator20/4
  VINP_CANMsgHMI2CommuOk_flg/1 -> Logical Operator20/5
  VINP_CANMsgVCU1RolCntOk_flg/1 -> Logical Operator20/6
  VINP_CANMsgVCU2RolCntOk_flg/1 -> Logical Operator20/7
  VINP_CANMsgHCU3RolCntOk_flg/1 -> Logical Operator20/8
  VINP_CANMsgVCU1ChkSumOk_flg/1 -> Logical Operator20/9
  VINP_CANMsgVCU2ChkSumOk_flg/1 -> Logical Operator20/10
  VINP_CANMsgEPAS1CommuOk_flg/1 -> Logical Operator7/2
  VINP_CANMsgEPAS2CommuOk_flg/1 -> Logical Operator7/3
  VINP_CANMsgEPAS3CommuOk_flg/1 -> Logical Operator7/4
  VINP_CANMsgSAS1RolCntOk_flg/1 -> Logical Operator7/5
  VINP_CANMsgEPAS2RolCntOk_flg/1 -> Logical Operator7/6
  VINP_CANMsgEPAS3RolCntOk_flg/1 -> Logical Operator7/7
  VINP_CANMsgSAS1ChkSumOk_flg/1 -> Logical Operator7/8
  VINP_CANMsgEPAS2ChkSumOk_flg/1 -> Logical Operator7/9
  VINP_CANMsgEPAS3ChkSumOk_flg/1 -> Logical Operator7/10
  Logical Operator36/1 -> Terminator11/1  "VINP_CSWCANInvalidFault_flg"
  Logical Operator35/1 -> Switch12/2
  Logical Operator34/1 -> Switch12/1
  ovrdflg11/1 -> Logical Operator35/1
  Switch12/1 -> Logical Operator36/1
  VINP_CANMsgCSW1CommuOk_flg/1 -> Logical Operator34/1
  ovrdval11/1 -> Switch12/3
  VINP_CANMsgEPMCommuOk_flg/1 -> Bus Selector/1
  Bus Selector/1 -> Logical Operator33/1  "<VINP_CANMsgEPM1CommuOk_flg>"
  Bus Selector/2 -> Logical Operator33/2  "<VINP_CANMsgEPM2CommuOk_flg>"
  Bus Selector/3 -> Logical Operator33/3  "<VINP_CANMsgEPM3CommuOk_flg>"
  Bus Selector/4 -> Logical Operator33/4  "<VINP_CANMsgEPM4CommuOk_flg>"
  Bus Selector/5 -> Logical Operator33/5  "<VINP_CANMsgEPM5CommuOk_flg>"
  Bus Selector/6 -> Logical Operator33/6  "<VINP_CANMsgEPM6CommuOk_flg>"
  Bus Selector/7 -> Logical Operator33/7  "<VINP_CANMsgEPM7CommuOk_flg>"
  Bus Selector/8 -> Logical Operator33/8  "<VINP_CANMsgEPM8CommuOk_flg>"
  Bus Selector/9 -> Logical Operator33/9  "<VINP_CANMsgEPM9CommuOk_flg>"
  Bus Selector/10 -> Logical Operator33/10  "<VINP_CANMsgEPM10CommuOk_flg>"
  Bus Selector/11 -> Logical Operator33/11  "<VINP_CANMsgEPM11CommuOk_flg>"
  Bus Selector/12 -> Logical Operator33/12  "<VINP_CANMsgEPM12CommuOk_flg>"
  Bus Selector/13 -> Logical Operator33/13  "<VINP_CANMsgEPM13CommuOk_flg>"
  Bus Selector/14 -> Logical Operator33/14  "<VINP_CANMsgEPM14CommuOk_flg>"
  Bus Selector/15 -> Logical Operator33/15  "<VINP_CANMsgEPM15CommuOk_flg>"
  Bus Selector/16 -> Logical Operator33/16  "<VINP_CANMsgEPM16CommuOk_flg>"
  Bus Selector/17 -> Logical Operator33/17  "<VINP_CANMsgEPM17CommuOk_flg>"
  Bus Selector/18 -> Logical Operator33/18  "<VINP_CANMsgEPM18CommuOk_flg>"
  Bus Selector/19 -> Logical Operator33/19  "<VINP_CANMsgEPM19CommuOk_flg>"
  Bus Selector/20 -> Logical Operator33/20  "<VINP_CANMsgEPM20CommuOk_flg>"
  Bus Selector/21 -> Logical Operator33/21  "<VINP_CANMsgEPM21CommuOk_flg>"
  Bus Selector/22 -> Logical Operator33/22  "<VINP_CANMsgEPM22CommuOk_flg>"
  Bus Selector/23 -> Logical Operator33/23  "<VINP_CANMsgEPM23CommuOk_flg>"
  Bus Selector/24 -> Logical Operator33/24  "<VINP_CANMsgEPM24CommuOk_flg>"
  Bus Selector/25 -> Logical Operator33/25  "<VINP_CANMsgEPM25CommuOk_flg>"
  Bus Selector/26 -> Logical Operator33/26  "<VINP_CANMsgEPM26CommuOk_flg>"
  Bus Selector/27 -> Logical Operator33/27  "<VINP_CANMsgEPM27CommuOk_flg>"
  Bus Selector/28 -> Logical Operator33/28  "<VINP_CANMsgEPM28CommuOk_flg>"
  Bus Selector/29 -> Logical Operator33/29  "<VINP_CANMsgEPM29CommuOk_flg>"
  Bus Selector/30 -> Logical Operator33/30  "<VINP_CANMsgEPM30CommuOk_flg>"
  Bus Selector/31 -> Logical Operator33/31  "<VINP_CANMsgEPM31CommuOk_flg>"
  Bus Selector/32 -> Logical Operator33/32  "<VINP_CANMsgEPM32CommuOk_flg>"
  Bus Selector/33 -> Logical Operator33/33  "<VINP_CANMsgEPM33CommuOk_flg>"
  Bus Selector/34 -> Logical Operator33/34  "<VINP_CANMsgEPM34CommuOk_flg>"
  Bus Selector/35 -> Logical Operator33/35  "<VINP_CANMsgEPM35CommuOk_flg>"
  VINP_CamCANReceiveOk_flg/1 -> Bus Selector1/1
  Bus Selector1/2 -> Logical Operator33/37  "<VINP_CamRightLaneReceiveOk_flg>"
  Bus Selector1/1 -> Logical Operator33/36  "<VINP_CamLeftLaneReceiveOk_flg>"
  Bus Selector1/3 -> Logical Operator33/38  "<VINP_CamObs1ReceiveOk_flg>"
  Bus Selector1/4 -> Logical Operator33/39  "<VINP_CamObs2ReceiveOk_flg>"
  Bus Selector1/5 -> Logical Operator33/40  "<VINP_CamObs3ReceiveOk_flg>"
  Bus Selector1/6 -> Logical Operator33/41  "<VINP_CamObs4ReceiveOk_flg>"
  Bus Selector1/7 -> Logical Operator33/42  "<VINP_CamObs5ReceiveOk_flg>"
  Bus Selector1/8 -> Logical Operator33/43  "<VINP_CamObs6ReceiveOk_flg>"
  Bus Selector1/9 -> Logical Operator33/44  "<VINP_CamObs7ReceiveOk_flg>"
  Bus Selector1/10 -> Logical Operator33/45  "<VINP_CamObs8ReceiveOk_flg>"
  Bus Selector1/11 -> Logical Operator33/46  "<VINP_CamObs9ReceiveOk_flg>"
  Bus Selector1/12 -> Logical Operator33/47  "<VINP_CamObs10ReceiveOk_flg>"
